simulink model "TosAeb"  (R2021a)
settings: Solver=FixedStepDiscrete  StartTime=0.0  StopTime=inf  FixedStep=0.01  RelTol=1e-3
undefined symbols (not defined in the file or in its folder): ACCModSts, AccSts1, AEB_ObjectMovingOrientation, AEB_ObjectMovingState, CN_BTCActive, CN_CurrentGearR, CN_CurrentGearR_DHT, CN_PTCActive, CN_VDCActive, CN_VSC_EspOff, CN_VSC_FCW_AEB_Error, CN_VSC_FCW_AEB_NoDispay, CN_VSC_FCW_AEB_PerfmDgdtn, CN_VSC_FCW_AEB_TempUnAvlbl, CN_VSC_VehStandstill, CNaccelAvoidMax, CNaccelAvoidMin, CNbrakePedalPress, CNcomfortStrLeftAccelMax, CNcomfortStrLeftAccelMin, CNcomfortStrRightAccelMax, CNcomfortStrRightAccelMin, CNDrvDoorOpen, CNDrvModeEco, CNDrvModeNorm, CNDrvModeOther, CNDrvModeSport, CNDrvSBRreminder, CNNull, CNobjInvalidID, CNPassDoorOpen, CNPassSBRreminder, CNPEBSAccelNegInf, CNPEBSAccelNull, CNPEBSTimeInf, CNPEBSTimeNull, CNSTOPMAX, CNSTOPNULL, CNsubjWidth, CNSwtOFF, CNSwtON, CNTTCMAX, CNTTCNULL, CNVehConfigAEBCycNone, CNVehConfigAEBNone, CNVehConfigAEBPedNone, CNVehConfigFCWNone, CNVehConfigJANone, CollisionSeverity, FALSE, FCWSnvty, JACycObjCalcInfoDefaultParams, JAPedObjCalcInfoDefaultParams, JAVehObjCalcInfoDefaultParams, latCycObjCalcInfoDefaultParams, latPedObjCalcInfoDefaultParams, longObjCalcInfoDefaultParams, ModSts, movingStatus, ObjectType ... (+1112)
libraries: simulink x3031, AEBSFunctionLib x50, Ta_Library x33, AEBSStateMachineLib x23, Mylibrary x12, simulink_extras x7, ObjSelect_lib x3, dspstat3 x2
(part 95 of 102)

== matlab function TosAeb/AEB_Objsel/TA_Processor/PreProcessor/ObjectPrecessor/TAObjInfoCalc/For Each Subsystem/Info_Check1/Orientation_check/Orientation_Classify ==
  data: ID_Change input; Fus_ObjOrientation output; Fus_ObsType input; Obj_Movingstate input; ObsID_u8 input; ObsMotionCategory_u8 input; ObsPedOrientation_u8 input; ObsPosX_s input; ObsPosY_s input; ObsVelY_s input; ObsVelXAbs_s input; ObsVelYAbs_s input; ObsCutInFlg_u8 input; ObsCutInSpd_s input; ObsCutInSpdValid_b input; ObsAngle_s input; ObsPosType_u8 input; ObsPosYDelay input; ObsSpdSqrt input; Obs_Vyaverage input
function Fus_ObjOrientation = fcn(ID_Change,Fus_ObsType,Obj_Movingstate,ObsID_u8,ObsMotionCategory_u8,ObsPedOrientation_u8,ObsPosX_s,ObsPosY_s,ObsVelY_s,...
    ObsVelXAbs_s,ObsVelYAbs_s,ObsCutInFlg_u8,ObsCutInSpd_s,ObsCutInSpdValid_b,ObsAngle_s,ObsPosType_u8,ObsPosYDelay,ObsSpdSqrt,Obs_Vyaverage)
%Fus_ObjOrientation 目标运动方向，定义如下
% 0：unknown 1:Preceeding 2:Crossing_to_Left
% 3: Crossing_to_Right 4:Cut_in 5:Cut_Out
% 6: Oncoming 7: LTAP 8:RTAP
ObjOrientation = uint8(0);
%检查目标是否为有效目标
if ID_Change == true || ObsID_u8 == uint8(0) ||Obj_Movingstate == uint8(0)||Obj_Movingstate == uint8(2)||Obj_Movingstate == uint8(3)
    ObjOrientation = uint8(0);
else
    %目标类型为车辆时目标的运动方向划分
    if Fus_ObsType > uint8(0) && Fus_ObsType < uint8(9)
        %Preceding判断
        if ObsPosX_s > single(0) && abs(ObsPosY_s) <= single(10.5) && ObsVelXAbs_s >= single(-0.5) && abs(ObsVelYAbs_s) <= (abs(ObsVelXAbs_s)+0.5) && (abs(ObsAngle_s)<single(pi/4)||abs(ObsAngle_s)>single(0.75*pi)) &&...
                ((ObsMotionCategory_u8 >= uint8(2) && ObsMotionCategory_u8 <= uint8(7))|| ObsMotionCategory_u8 == uint8(12))&& ObsPosType_u8>= uint8(1) && ObsPosType_u8<= uint8(3)
            %cut_in cut_out 判断
            if (ObsMotionCategory_u8 == uint8(5)||ObsMotionCategory_u8 == uint8(6)) && ObsCutInFlg_u8 == uint8(1) && abs(ObsCutInSpd_s) > single(0) && ObsCutInSpdValid_b == true && abs(ObsVelYAbs_s)>single(0)
                ObjOrientation = uint8(4);
            elseif ObsMotionCategory_u8 == uint8(7) && abs(ObsPosYDelay) - abs(ObsPosY_s) > single(0)
                ObjOrientation = uint8(5);
            else
                ObjOrientation = uint8(1);
            end
            % Crossing_to_Left/Right判断
        elseif ObsPosX_s > single(0) && ObsVelYAbs_s>= single(0) && abs(ObsVelYAbs_s) >= abs((ObsVelXAbs_s)+0.5) && (abs(ObsAngle_s)>single(pi/4)&&abs(ObsAngle_s)<single(0.75*pi))&& ObsMotionCategory_u8 == uint8(12)
            if ObsVelY_s > single(0) && ObsPosType_u8 ~= uint8(7) && ObsPosYDelay - ObsPosY_s< single(0)
                ObjOrientation = uint8(2);
            elseif ObsVelY_s < single(0) && ObsPosType_u8 ~= uint8(8) && ObsPosYDelay - ObsPosY_s> single(0)
                ObjOrientation = uint8(3);
            end
            % Oncoming
        elseif ObsPosX_s > single(0) && abs(ObsPosY_s) <= single(10.5) && ObsVelXAbs_s<single(0) && abs(ObsVelYAbs_s) <= abs(ObsVelXAbs_s) && (abs(ObsAngle_s)<single(pi/4)||abs(ObsAngle_s)>single(0.75*pi)) &&...
                ObsMotionCategory_u8 == uint8(13) && ObsPosType_u8 >= uint8(4) && ObsPosType_u8<= uint8(6)
            ObjOrientation = uint8(6);
            % LTAP
        elseif ObsMotionCategory_u8 == uint8(9)
            ObjOrientation = uint8(7);
            % RTAP
        elseif ObsMotionCategory_u8 == uint8(10)
            ObjOrientation = uint8(8);
        else
            ObjOrientation = uint8(0);
        end
        %二轮车运动方向判断
    elseif Fus_ObsType == uint8(9)||Fus_ObsType == uint8(10)
        if ObsPosX_s > single(0) && abs(ObsPosY_s) <= single(10.5) && ObsVelXAbs_s >= single(-0.5) && abs(ObsVelYAbs_s) <= (abs(ObsVelXAbs_s)+0.5) && (abs(ObsAngle_s)<single(pi/4)||abs(ObsAngle_s)>single(0.75*pi))
            %cut_in cut_out 判断
            if  abs(ObsVelYAbs_s)>single(0.5)&&abs(ObsVelYAbs_s)<single(2)&&ObsVelXAbs_s>ObsVelYAbs_s&&abs(ObsPosYDelay) - abs(ObsPosY_s) < single(0)
                ObjOrientation = uint8(4);
            elseif abs(ObsVelYAbs_s)>single(0.5)&&abs(ObsVelYAbs_s)<single(2)&&ObsVelXAbs_s>ObsVelYAbs_s&&abs(ObsPosYDelay) - abs(ObsPosY_s) > single(0)
                ObjOrientation = uint8(5);
            else
                ObjOrientation = uint8(1);
            end
            % Crossing_to_Left/Right判断
        elseif ObsPosX_s > single(0) && ObsVelYAbs_s>= single(0) && abs(ObsVelYAbs_s) >= abs((ObsVelXAbs_s)+0.5) && (abs(ObsAngle_s)>single(pi/4)&&abs(ObsAngle_s)<single(0.75*pi))
            if ObsVelY_s > single(0) && ObsPosYDelay - ObsPosY_s< single(0)
                ObjOrientation = uint8(3);
            elseif ObsVelY_s < single(0) && ObsPosYDelay - ObsPosY_s> single(0)
                ObjOrientation = uint8(2);
            end
            % Oncoming
        elseif ObsPosX_s > single(0) && abs(ObsPosY_s) <= single(10.5) && ObsVelXAbs_s<single(0) && abs(ObsVelYAbs_s) <= abs(ObsVelXAbs_s) && (abs(ObsAngle_s)<single(pi/4)||abs(ObsAngle_s)>single(0.75*pi))
            ObjOrientation = uint8(6);
            % LTAP
        elseif (abs(ObsAngle_s)>single(pi/4)&&abs(ObsAngle_s)<single(0.75*pi))&&ObsVelXAbs_s<single(-0.5)&&ObsVelYAbs_s<single(-0.5)
            ObjOrientation = uint8(7);
            % RTAP
        elseif (abs(ObsAngle_s)>single(pi/4)&&abs(ObsAngle_s)<single(0.75*pi))&&ObsVelXAbs_s<single(-0.5)&&ObsVelYAbs_s>single(-0.5)
            ObjOrientation = uint8(8);
        else
            ObjOrientation = uint8(0);
        end  
        %目标类型为行人或两轮车时目标的运动方向划分
    else
        if (ObsPedOrientation_u8 == uint8(1)||ObsPedOrientation_u8 == uint8(5)||ObsPedOrientation_u8 == uint8(8))&& ObsPosX_s > single(0)
            %Oncoming
            if ObsVelXAbs_s < single(-1) && abs(ObsVelYAbs_s) <= (abs(ObsVelXAbs_s)) && abs(ObsPosYDelay) - abs(ObsPosY_s) < single(1) && ObsSpdSqrt>=single(0.5)
                ObjOrientation = uint8(6);
                %Crossing_to_Left/Right判断
            elseif (abs(ObsVelYAbs_s) >= (abs(ObsVelXAbs_s)) || abs(ObsPosYDelay) - abs(ObsPosY_s) >= single(0.05)) && ObsVelY_s >single(0) && ObsPosYDelay - ObsPosY_s < single(0)&& abs(ObsVelY_s) >single(0.5)
                ObjOrientation = uint8(3);
            elseif (abs(ObsVelYAbs_s) >= (abs(ObsVelXAbs_s)) || abs(ObsPosYDelay) - abs(ObsPosY_s) >= single(0.05)) && ObsVelY_s <single(0) && ObsPosYDelay - ObsPosY_s > single(0)&& abs(ObsVelY_s) >single(0.5)
                ObjOrientation = uint8(2);
            elseif abs(ObsVelY_s) <= single(0.5)|| ObsSpdSqrt<=single(0.5)
                ObjOrientation = uint8(1);
            end
        elseif (ObsPedOrientation_u8 == uint8(2)||ObsPedOrientation_u8 == uint8(4)||ObsPedOrientation_u8 == uint8(7))&& ObsPosX_s > single(0)
            if ObsVelXAbs_s < single(-1) && abs(ObsVelYAbs_s) <= (abs(ObsVelXAbs_s)) && abs(ObsPosYDelay) - abs(ObsPosY_s) < single(0.05) && ObsSpdSqrt>=single(0.5)
                ObjOrientation = uint8(6);
                %Crossing_to_Left/Right判断
            elseif ObsVelXAbs_s > single(-0.5) && (abs(ObsVelYAbs_s) >= (abs(ObsVelXAbs_s)) || abs(ObsPosYDelay) - abs(ObsPosY_s) >= single(0.25)) && ObsVelY_s < single(0) && ObsPosYDelay - ObsPosY_s > single(0)
                ObjOrientation = uint8(3);
            elseif ObsVelXAbs_s > single(-0.5) && (abs(ObsVelYAbs_s) >= (abs(ObsVelXAbs_s)) || abs(ObsPosYDelay) - abs(ObsPosY_s) >= single(0.25)) && ObsVelY_s > single(0) && ObsPosYDelay - ObsPosY_s < single(0)
                ObjOrientation = uint8(2);
            elseif abs(ObsVelY_s) <= single(0.5)|| ObsSpdSqrt<=single(0.5)
                ObjOrientation = uint8(1);
            end
        elseif (ObsPedOrientation_u8 == uint8(3)||ObsPedOrientation_u8 == uint8(6))&& ObsPosX_s > single(0)
            if ObsVelXAbs_s < single(-1) && abs(ObsVelYAbs_s) <= (abs(ObsVelXAbs_s)) && abs(ObsPosYDelay) - abs(ObsPosY_s) < single(1) && ObsSpdSqrt>=single(0.5)
                ObjOrientation = uint8(6);
                %Crossing_to_Left/Right判断
            elseif(abs(ObsVelYAbs_s) >= (abs(ObsVelXAbs_s)) || abs(ObsPosYDelay) - abs(ObsPosY_s) >= single(0.05)) && ObsVelY_s < single(0) && ObsPosYDelay - ObsPosY_s > single(0) &&abs(Obs_Vyaverage)>single(0.5)
                ObjOrientation = uint8(3);
            elseif (abs(ObsVelYAbs_s) >= (abs(ObsVelXAbs_s)) || abs(ObsPosYDelay) - abs(ObsPosY_s) >= single(0.05)) && ObsVelY_s > single(0) && ObsPosYDelay - ObsPosY_s < single(0) && abs(Obs_Vyaverage)>single(0.5)
                ObjOrientation = uint8(2);
            elseif abs(ObsVelY_s) <= abs(ObsVelXAbs_s)|| ObsSpdSqrt<=single(0.5)
                ObjOrientation = uint8(1);
            end
        else
            ObjOrientation = uint8(0);
        end
    end
end
Fus_ObjOrientation = ObjOrientation;
end

== matlab function TosAeb/AEB_Objsel/TA_Processor/PreProcessor/ObjectPrecessor/TAObjInfoCalc/For Each Subsystem/Info_Check1/Orientation_check/Orientation_Classify2 ==
  data: ID_Change input; Fus_ObjOrientation output; Fus_ObsType input; ObsID_u8 input; ObsMotionCategory_u8 input; ObsPedOrientation_u8 input; ObsPosX_s input; ObsPosY_s input; ObsVelY_s input; ObsVelXAbs_s input; ObsVelYAbs_s input; ObsCutInFlg_u8 input; ObsCutInSpd_s input; ObsCutInSpdValid_b input; ObsAngle_s input; ObsPosType_u8 input; ObsPosYDelay input; ObsSpdSqrt input
function Fus_ObjOrientation = fcn(ID_Change,Fus_ObsType,ObsID_u8,ObsMotionCategory_u8,ObsPedOrientation_u8,ObsPosX_s,ObsPosY_s,ObsVelY_s,...
    ObsVelXAbs_s,ObsVelYAbs_s,ObsCutInFlg_u8,ObsCutInSpd_s,ObsCutInSpdValid_b,ObsAngle_s,ObsPosType_u8,ObsPosYDelay,ObsSpdSqrt)
%Fus_ObjOrientation 目标运动方向，定义如下
% 0：unknown 1:Preceeding 2:Crossing_to_Left
% 3: Crossing_to_Right 4:Cut_in 5:Cut_Out
% 6: Oncoming 7: LTAP 8:RTAP
ObjOrientation = uint8(0);
%检查目标是否为有效目标
if ID_Change == true || ObsID_u8 == uint8(0)
    ObjOrientation = uint8(0);
else
    %目标类型为车辆时目标的运动方向划分
    if Fus_ObsType > uint8(0) && Fus_ObsType < uint8(9)
        %Preceding判断
        if ObsPosX_s > single(0) && abs(ObsPosY_s) <= single(10.5) && ObsVelXAbs_s >= single(-0.5) && abs(ObsVelYAbs_s) <= (abs(ObsVelXAbs_s)+0.5) && (abs(ObsAngle_s)<single(pi/4)||abs(ObsAngle_s)>single(0.75*pi)) &&...
                ((ObsMotionCategory_u8 >= uint8(2) && ObsMotionCategory_u8 <= uint8(7))|| ObsMotionCategory_u8 == uint8(12))&& ObsPosType_u8>= uint8(1) && ObsPosType_u8<= uint8(3)
            %cut_in cut_out 判断
            if (ObsMotionCategory_u8 == uint8(5)||ObsMotionCategory_u8 == uint8(6)) && ObsCutInFlg_u8 == uint8(1) && abs(ObsCutInSpd_s) > single(0) && ObsCutInSpdValid_b == true && abs(ObsVelYAbs_s)>single(0)
                ObjOrientation = uint8(4);
            elseif ObsMotionCategory_u8 == uint8(7) && abs(ObsPosYDelay) - abs(ObsPosY_s) > single(0)
                ObjOrientation = uint8(5);
            else
                ObjOrientation = uint8(1);
            end
            % Crossing_to_Left/Right判断
        elseif ObsPosX_s > single(0) && abs(ObsVelYAbs_s) >= single(0) && abs(ObsVelYAbs_s) >= abs((ObsVelXAbs_s)+0.5) && (abs(ObsAngle_s)>single(pi/4)&&abs(ObsAngle_s)<single(0.75*pi))&& ObsMotionCategory_u8 == uint8(12)
            if ObsVelY_s > single(0) && ObsPosType_u8 ~= uint8(7) && ObsPosYDelay - ObsPosY_s< single(0)
                ObjOrientation = uint8(2);
            elseif ObsVelY_s < single(0) && ObsPosType_u8 ~= uint8(8) && ObsPosYDelay - ObsPosY_s> single(0)
                ObjOrientation = uint8(3);
            end
            % Oncoming
        elseif ObsPosX_s > single(0) && abs(ObsPosY_s) <= single(10.5) && ObsVelXAbs_s<single(0) && abs(ObsVelYAbs_s) <= abs(ObsVelXAbs_s) && (abs(ObsAngle_s)<single(pi/4)||abs(ObsAngle_s)>single(0.75*pi)) &&...
                ObsMotionCategory_u8 == uint8(13) && ObsPosType_u8 >= uint8(4) && ObsPosType_u8<= uint8(6)
            ObjOrientation = uint8(6);
            % LTAP
        elseif ObsMotionCategory_u8 == uint8(9)
            ObjOrientation = uint8(7);
            % RTAP
        elseif ObsMotionCategory_u8 == uint8(10)
            ObjOrientation = uint8(8);
        else
            ObjOrientation = uint8(0);
        end
        %二轮车运动方向判断
    elseif Fus_ObsType == uint8(9)||Fus_ObsType == uint8(10)
        if ObsPosX_s > single(0) && abs(ObsPosY_s) <= single(10.5) && ObsVelXAbs_s >= single(-0.5) && abs(ObsVelYAbs_s) <= (abs(ObsVelXAbs_s)+0.5) && (abs(ObsAngle_s)<single(pi/4)||abs(ObsAngle_s)>single(0.75*pi))
            %cut_in cut_out 判断
            if  abs(ObsVelYAbs_s)>single(0.5)&&abs(ObsVelYAbs_s)<single(2)&&ObsVelXAbs_s>ObsVelYAbs_s&&abs(ObsPosYDelay) - abs(ObsPosY_s) < single(0)
                ObjOrientation = uint8(4);
            elseif abs(ObsVelYAbs_s)>single(0.5)&&abs(ObsVelYAbs_s)<single(2)&&ObsVelXAbs_s>ObsVelYAbs_s&&abs(ObsPosYDelay) - abs(ObsPosY_s) > single(0)
                ObjOrientation = uint8(5);
            else
                ObjOrientation = uint8(1);
            end
            % Crossing_to_Left/Right判断
        elseif ObsPosX_s > single(0) && abs(ObsVelYAbs_s) >= single(0) && abs(ObsVelYAbs_s) >= abs((ObsVelXAbs_s)+0.5) && abs(ObsAngle_s)>single(pi/4) && abs(ObsAngle_s)<single(0.75*pi)
            if ObsVelY_s > single(0) && ObsPosYDelay - ObsPosY_s< single(0)
                ObjOrientation = uint8(2);
            elseif ObsVelY_s < single(0) && ObsPosYDelay - ObsPosY_s> single(0)
                ObjOrientation = uint8(3);
            end
            % Oncoming
        elseif ObsPosX_s > single(0) && abs(ObsPosY_s) <= single(10.5) && ObsVelXAbs_s<single(0) && abs(ObsVelYAbs_s) <= abs(ObsVelXAbs_s) && (abs(ObsAngle_s)<single(pi/4)||abs(ObsAngle_s)>single(0.75*pi))
            ObjOrientation = uint8(6);
            % LTAP
        elseif (abs(ObsAngle_s)>single(pi/4)&&abs(ObsAngle_s)<single(0.75*pi))&&ObsVelXAbs_s<single(-0.5)&&ObsVelYAbs_s<single(-0.5)
            ObjOrientation = uint8(7);
            % RTAP
        elseif (abs(ObsAngle_s)>single(pi/4)&&abs(ObsAngle_s)<single(0.75*pi))&&ObsVelXAbs_s<single(-0.5)&&ObsVelYAbs_s>single(-0.5)
            ObjOrientation = uint8(8);
        else
            ObjOrientation = uint8(0);
        end  
        %目标类型为行人或两轮车时目标的运动方向划分
    else
        if (ObsPedOrientation_u8 == uint8(1)||ObsPedOrientation_u8 == uint8(5)||ObsPedOrientation_u8 == uint8(8))&& ObsPosX_s > single(0)
            %Oncoming
            if ObsVelXAbs_s < single(-1) && abs(ObsVelYAbs_s) <= (abs(ObsVelXAbs_s)+single(0.5)) && abs(ObsPosYDelay) - abs(ObsPosY_s) < single(1) && ObsSpdSqrt>=single(0.5)
                ObjOrientation = uint8(6);
                %Crossing_to_Left/Right判断
            elseif ObsVelXAbs_s > single(-0.5) && (abs(ObsVelYAbs_s) >= (abs(ObsVelXAbs_s)+single(0.5)) || abs(ObsPosYDelay) - abs(ObsPosY_s) >= single(1)) && ObsVelY_s >single(0) && ObsPosYDelay - ObsPosY_s < single(0)&& abs(ObsVelY_s) >single(0.5)
                ObjOrientation = uint8(2);
            elseif ObsVelXAbs_s > single(-0.5) && (abs(ObsVelYAbs_s) >= (abs(ObsVelXAbs_s)+single(0.5)) || abs(ObsPosYDelay) - abs(ObsPosY_s) >= single(1)) && ObsVelY_s <single(0) && ObsPosYDelay - ObsPosY_s > single(0)&& abs(ObsVelY_s) >single(0.5)
                ObjOrientation = uint8(3);
            elseif abs(ObsVelY_s) <= single(0.5)|| ObsSpdSqrt<=single(0.5)
                ObjOrientation = uint8(1);
            end
        elseif (ObsPedOrientation_u8 == uint8(2)||ObsPedOrientation_u8 == uint8(4)||ObsPedOrientation_u8 == uint8(7))&& ObsPosX_s > single(0)
            if ObsVelXAbs_s < single(-1) && abs(ObsVelYAbs_s) <= (abs(ObsVelXAbs_s)+single(0.5)) && abs(ObsPosYDelay) - abs(ObsPosY_s) < single(1) && ObsSpdSqrt>=single(0.5)
                ObjOrientation = uint8(6);
                %Crossing_to_Left/Right判断
            elseif ObsVelXAbs_s > single(-0.5) && (abs(ObsVelYAbs_s) >= (abs(ObsVelXAbs_s)+single(0.5)) || abs(ObsPosYDelay) - abs(ObsPosY_s) >= single(1)) && ObsVelY_s >single(0) && ObsPosYDelay - ObsPosY_s < single(0)&& abs(ObsVelY_s) >single(0.5)
                ObjOrientation = uint8(2);
            elseif ObsVelXAbs_s > single(-0.5) && (abs(ObsVelYAbs_s) >= (abs(ObsVelXAbs_s)+single(0.5)) || abs(ObsPosYDelay) - abs(ObsPosY_s) >= single(1)) && ObsVelY_s <single(0) && ObsPosYDelay - ObsPosY_s > single(0)&& abs(ObsVelY_s) >single(0.5)
                ObjOrientation = uint8(3);
            elseif abs(ObsVelY_s) <= single(0.5)|| ObsSpdSqrt<=single(0.5)
                ObjOrientation = uint8(1);
            end
        elseif (ObsPedOrientation_u8 == uint8(3)||ObsPedOrientation_u8 == uint8(6))&& ObsPosX_s > single(0)
            if ObsVelXAbs_s < single(-1) && abs(ObsVelYAbs_s) <= (abs(ObsVelXAbs_s)+single(0.5)) && abs(ObsPosYDelay) - abs(ObsPosY_s) < single(1) && ObsSpdSqrt>=single(0.5)
                ObjOrientation = uint8(6);
                 %Crossing_to_Left/Right判断
            elseif ObsVelXAbs_s > single(-0.5) && (abs(ObsVelYAbs_s) >= (abs(ObsVelXAbs_s)+single(0.5)) || abs(ObsPosYDelay) - abs(ObsPosY_s) >= single(1)) && ObsVelY_s >single(0) && ObsPosYDelay - ObsPosY_s < single(0)&& abs(ObsVelY_s) >single(0.5)
                ObjOrientation = uint8(2);
            elseif ObsVelXAbs_s > single(-0.5) && (abs(ObsVelYAbs_s) >= (abs(ObsVelXAbs_s)+single(0.5)) || abs(ObsPosYDelay) - abs(ObsPosY_s) >= single(1)) && ObsVelY_s <single(0) && ObsPosYDelay - ObsPosY_s > single(0)&& abs(ObsVelY_s) >single(0.5)
                ObjOrientation = uint8(3);
            elseif abs(ObsVelY_s) <= single(0.5)|| ObsSpdSqrt<=single(0.5)
                ObjOrientation = uint8(1);
            end
        else
            ObjOrientation = uint8(0);
        end
    end
end
Fus_ObjOrientation = ObjOrientation;
end

== matlab function TosAeb/AEB_Objsel/TA_Processor/PreProcessor/ObjectPrecessor/TAObjInfoCalc/For Each Subsystem/Info_Check1/Orientation_check/Orientation_Classify3 ==
  data: ID_Change input; Fus_ObjOrientation output; Fus_ObsType input; ObsID_u8 input; ObsMotionCategory_u8 input; ObsPedOrientation_u8 input; ObsPosX_s input; ObsPosY_s input; ObsVelY_s input; ObsVelXAbs_s input; ObsVelYAbs_s input; ObsCutInFlg_u8 input; ObsCutInSpd_s input; ObsCutInSpdValid_b input; ObsAngle_s input; ObsPosType_u8 input; ObsPosYDelay input; ObsSpdSqrt input
function Fus_ObjOrientation = fcn(ID_Change,Fus_ObsType,ObsID_u8,ObsMotionCategory_u8,ObsPedOrientation_u8,ObsPosX_s,ObsPosY_s,ObsVelY_s,...
    ObsVelXAbs_s,ObsVelYAbs_s,ObsCutInFlg_u8,ObsCutInSpd_s,ObsCutInSpdValid_b,ObsAngle_s,ObsPosType_u8,ObsPosYDelay,ObsSpdSqrt)
%Fus_ObjOrientation 目标运动方向，定义如下
% 0：unknown 1:Preceeding 2:Crossing_to_Left
% 3: Crossing_to_Right 4:Cut_in 5:Cut_Out
% 6: Oncoming 7: LTAP 8:RTAP
ObjOrientation = uint8(0);
%检查目标是否为有效目标
if ID_Change == true || ObsID_u8 == uint8(0)
    ObjOrientation = uint8(0);
else
    %目标类型为车辆时目标的运动方向划分
    if Fus_ObsType > uint8(0) && Fus_ObsType < uint8(9)
        %Preceding判断
        if ObsPosX_s > single(0) && abs(ObsPosY_s) <= single(10.5) && ObsVelXAbs_s >= single(-0.5) && abs(ObsVelYAbs_s) <= (abs(ObsVelXAbs_s)+0.5) && (abs(ObsAngle_s)<single(pi/4)||abs(ObsAngle_s)>single(0.75*pi)) &&...
                ((ObsMotionCategory_u8 >= uint8(2) && ObsMotionCategory_u8 <= uint8(7))|| ObsMotionCategory_u8 == uint8(12))
            %cut_in cut_out 判断
            if (ObsMotionCategory_u8 == uint8(5)||ObsMotionCategory_u8 == uint8(6)) && ObsCutInFlg_u8 == uint8(1) && abs(ObsCutInSpd_s) > single(0) && ObsCutInSpdValid_b == true && abs(ObsVelYAbs_s)>single(0)
                ObjOrientation = uint8(4);
            elseif ObsMotionCategory_u8 == uint8(7) && abs(ObsPosYDelay) - abs(ObsPosY_s) > single(0)
                ObjOrientation = uint8(5);
            else
                ObjOrientation = uint8(1);
            end
            % Crossing_to_Left/Right判断
        elseif ObsPosX_s > single(0) && abs(ObsVelYAbs_s) >= single(0) && abs(ObsVelYAbs_s) >= abs((ObsVelXAbs_s)+0.5) && (abs(ObsAngle_s)>single(pi/4)&&abs(ObsAngle_s)<single(0.75*pi))&& ObsMotionCategory_u8 == uint8(12)
            if ObsVelY_s > single(0) && ObsPosType_u8 ~= uint8(7) && ObsPosYDelay - ObsPosY_s< single(0)
                ObjOrientation = uint8(2);
            elseif ObsVelY_s < single(0) && ObsPosType_u8 ~= uint8(8) && ObsPosYDelay - ObsPosY_s> single(0)
                ObjOrientation = uint8(3);
            end
            % Oncoming
        elseif ObsPosX_s > single(0) && abs(ObsPosY_s) <= single(10.5) && ObsVelXAbs_s<single(0) && abs(ObsVelYAbs_s) <= abs(ObsVelXAbs_s) && (abs(ObsAngle_s)<single(pi/4)||abs(ObsAngle_s)>single(0.75*pi)) &&...
                ObsMotionCategory_u8 == uint8(13) && ObsPosType_u8 >= uint8(4) && ObsPosType_u8<= uint8(6)
            ObjOrientation = uint8(6);
            % LTAP
        elseif ObsMotionCategory_u8 == uint8(9)
            ObjOrientation = uint8(7);
            % RTAP
        elseif ObsMotionCategory_u8 == uint8(10)
            ObjOrientation = uint8(8);
        else
            ObjOrientation = uint8(0);
        end
        %二轮车运动方向判断
    elseif Fus_ObsType == uint8(9)||Fus_ObsType == uint8(10)
        if ObsPosX_s > single(0) && abs(ObsPosY_s) <= single(10.5) && ObsVelXAbs_s >= single(-0.5) && abs(ObsVelYAbs_s) <= (abs(ObsVelXAbs_s)+0.5) && (abs(ObsAngle_s)<single(pi/4)||abs(ObsAngle_s)>single(0.75*pi))
            %cut_in cut_out 判断
            if  abs(ObsVelYAbs_s)>single(0.5)&&abs(ObsVelYAbs_s)<single(2)&&ObsVelXAbs_s>ObsVelYAbs_s&&abs(ObsPosYDelay) - abs(ObsPosY_s) < single(0)
                ObjOrientation = uint8(4);
            elseif abs(ObsVelYAbs_s)>single(0.5)&&abs(ObsVelYAbs_s)<single(2)&&ObsVelXAbs_s>ObsVelYAbs_s&&abs(ObsPosYDelay) - abs(ObsPosY_s) > single(0)
                ObjOrientation = uint8(5);
            else
                ObjOrientation = uint8(1);
            end
            % Crossing_to_Left/Right判断
        elseif ObsPosX_s > single(0) % && abs(ObsVelYAbs_s) >= single(0) && abs(ObsVelYAbs_s) >= abs((ObsVelXAbs_s)+0.5) && abs(ObsAngle_s)>single(pi/4) && abs(ObsAngle_s)<single(0.75*pi) % 20240125
            if ObsVelXAbs_s > single(-0.5) && (abs(ObsVelYAbs_s) >= (abs(ObsVelXAbs_s)+single(0.5)) || abs(ObsPosYDelay) - abs(ObsPosY_s) >= single(0.05)) && ObsVelY_s >single(0) && ObsPosYDelay - ObsPosY_s < single(0)&& abs(ObsVelY_s) >single(0.5)
                %ObsVelY_s > single(0) && ObsPosYDelay - ObsPosY_s<single(0） 
                ObjOrientation = uint8(2);
            elseif ObsVelXAbs_s > single(-0.5) && (abs(ObsVelYAbs_s) >= (abs(ObsVelXAbs_s)+single(0.5)) || abs(ObsPosYDelay) - abs(ObsPosY_s) >= single(0.05)) && ObsVelY_s <single(0) && ObsPosYDelay - ObsPosY_s > single(0)&& abs(ObsVelY_s) >single(0.5)
                %ObsVelY_s < single(0) && ObsPosYDelay - ObsPosY_s> single(0)
                ObjOrientation = uint8(3);
            end
            % Oncoming
        elseif ObsPosX_s > single(0) && abs(ObsPosY_s) <= single(10.5) && ObsVelXAbs_s<single(0) && abs(ObsVelYAbs_s) <= abs(ObsVelXAbs_s) && (abs(ObsAngle_s)<single(pi/4)||abs(ObsAngle_s)>single(0.75*pi))
            ObjOrientation = uint8(6);
            % LTAP
        elseif (abs(ObsAngle_s)>single(pi/4)&&abs(ObsAngle_s)<single(0.75*pi))&&ObsVelXAbs_s<single(-0.5)&&ObsVelYAbs_s<single(-0.5)
            ObjOrientation = uint8(7);
            % RTAP
        elseif (abs(ObsAngle_s)>single(pi/4)&&abs(ObsAngle_s)<single(0.75*pi))&&ObsVelXAbs_s<single(-0.5)&&ObsVelYAbs_s>single(-0.5)
            ObjOrientation = uint8(8);
        else
            ObjOrientation = uint8(0);
        end  
        %目标类型为行人或两轮车时目标的运动方向划分
    else
        if (ObsPedOrientation_u8 == uint8(1)||ObsPedOrientation_u8 == uint8(5)||ObsPedOrientation_u8 == uint8(8))&& ObsPosX_s > single(0)
            %Oncoming
            if ObsVelXAbs_s < single(-1) && abs(ObsVelYAbs_s) <= (abs(ObsVelXAbs_s)+single(0.5)) && abs(ObsPosYDelay) - abs(ObsPosY_s) < single(1) && ObsSpdSqrt>=single(0.5)
                ObjOrientation = uint8(6);
                %Crossing_to_Left/Right判断
            elseif ObsVelXAbs_s > single(-0.5) && (abs(ObsVelYAbs_s) >= (abs(ObsVelXAbs_s)+single(0.5))&& ObsVelY_s >single(0))          % || abs(ObsPosYDelay) - abs(ObsPosY_s) >= single(0.5)) && ObsVelY_s >single(0) && ObsPosYDelay - ObsPosY_s < single(0)&& abs(ObsVelY_s) >single(0.5)
                ObjOrientation = uint8(2);
            elseif ObsVelXAbs_s > single(-0.5) && (abs(ObsVelYAbs_s) >= (abs(ObsVelXAbs_s)+single(0.5))&&ObsVelY_s <single(0))             %|| abs(ObsPosYDelay) - abs(ObsPosY_s) <= single(0.5)) && ObsVelY_s <single(0) && ObsPosYDelay - ObsPosY_s > single(0)&& abs(ObsVelY_s) >single(0.5)
                ObjOrientation = uint8(3);
            elseif abs(ObsVelY_s) <= single(0.5)|| ObsSpdSqrt<=single(0.5)
                ObjOrientation = uint8(1);
            end
        elseif (ObsPedOrientation_u8 == uint8(2)||ObsPedOrientation_u8 == uint8(4)||ObsPedOrientation_u8 == uint8(7))&& ObsPosX_s > single(0)
            if ObsVelXAbs_s < single(-1) && abs(ObsVelYAbs_s) <= (abs(ObsVelXAbs_s)+single(0.5)) && abs(ObsPosYDelay) - abs(ObsPosY_s) < single(1) && ObsSpdSqrt>=single(0.5)
                ObjOrientation = uint8(6);
                %Crossing_to_Left/Right判断
            elseif ObsVelXAbs_s > single(-0.5) && (abs(ObsVelYAbs_s) >= (abs(ObsVelXAbs_s)+single(0.5))&& ObsVelY_s >single(0)) %      || abs(ObsPosYDelay) - abs(ObsPosY_s) >= single(1)) && ObsVelY_s >single(0) && ObsPosYDelay - ObsPosY_s < single(0)&& abs(ObsVelY_s) >single(0.5)
                ObjOrientation = uint8(2);
            elseif ObsVelXAbs_s > single(-0.5) && (abs(ObsVelYAbs_s) >= (abs(ObsVelXAbs_s)+single(0.5))&& ObsVelY_s <single(0)) %      || abs(ObsPosYDelay) - abs(ObsPosY_s) >= single(1)) && ObsVelY_s <single(0) && ObsPosYDelay - ObsPosY_s > single(0)&& abs(ObsVelY_s) >single(0.5)
                ObjOrientation = uint8(3);
            elseif abs(ObsVelY_s) <= single(0.5)|| ObsSpdSqrt<=single(0.5)
                ObjOrientation = uint8(1);
            end
        elseif (ObsPedOrientation_u8 == uint8(3)||ObsPedOrientation_u8 == uint8(6))&& ObsPosX_s > single(0)
            if ObsVelXAbs_s < single(-1) && abs(ObsVelYAbs_s) <= (abs(ObsVelXAbs_s)+single(0.5)) && abs(ObsPosYDelay-ObsPosY_s) < single(0.5) && ObsSpdSqrt>=single(0.5)
                ObjOrientation = uint8(6);
                 %Crossing_to_Left/Right判断
            elseif ObsVelXAbs_s > single(-0.5) && (abs(ObsVelYAbs_s) >= (abs(ObsVelXAbs_s)+single(0.5)) && ObsVelY_s >single(0))
            %elseif ObsVelXAbs_s > single(-0.5) && (abs(ObsVelYAbs_s) >= (abs(ObsVelXAbs_s)+single(0.5)) || abs(ObsPosYDelay-ObsPosY_s) >= single(1)) && ObsVelY_s >single(0) && ObsPosYDelay - ObsPosY_s < single(0)&& abs(ObsVelY_s) >single(0.5)
                ObjOrientation = uint8(2);
            
            %elseif ObsVelXAbs_s > single(-0.5) && (abs(ObsVelYAbs_s) >= (abs(ObsVelXAbs_s)+single(0.5)) || abs(ObsPosYDelay- ObsPosY_s) >= single(1)) && ObsVelY_s <single(0) && ObsPosYDelay - ObsPosY_s > single(0)&& abs(ObsVelY_s) >single(0.5)
             elseif ObsVelXAbs_s > single(-0.5) && (abs(ObsVelYAbs_s) >= (abs(ObsVelXAbs_s)+single(0.5)) && ObsVelY_s <single(0))
            ObjOrientation = uint8(3);
            elseif abs(ObsVelY_s) <= single(0.5)|| ObsSpdSqrt<=single(0.5)
                ObjOrientation = uint8(1);
            end
        else
            ObjOrientation = uint8(0);
        end
    end
end
Fus_ObjOrientation = ObjOrientation;
end

== matlab function TosAeb/AEB_Objsel/TA_Processor/PreProcessor/ObjectPrecessor/TAObjInfoCalc/For Each Subsystem/Info_Check1/State_check/State_Classify1 ==
  data: ID_Change input; Fus_ObjState output; Fus_ObsType input; ObsID_u8 input; ObsMeasureStatus_u8 input; ObsVelXAbs_s input; ObsVelYAbs_s input; Spd_Stay input
function Fus_ObjState = fcn(ID_Change, Fus_ObsType, ObsID_u8, ObsMeasureStatus_u8, ObsVelXAbs_s, ObsVelYAbs_s, Spd_Stay)

%Fus_ObjState 目标运动状态
% 0：unknown 1:Moving 2:Stationary
% 3: Stopped 4:Moving_Slowly
ObjState = uint8(0);
%判断目标ID是否有效
if ID_Change == true || ObsID_u8 == uint8(0)
    ObjState = uint8(0);
else
    %对目标类型为车辆的运动状态进行校核
    if Fus_ObsType > uint8(0) && Fus_ObsType < uint8(9)
        if ObsMeasureStatus_u8 == uint8(1)
            if abs(ObsVelXAbs_s) + abs(ObsVelYAbs_s) > single(2.5)
                ObjState = uint8(1);
            elseif abs(ObsVelXAbs_s) + abs(ObsVelYAbs_s) <= single(0.9) && Spd_Stay <= single(0.5)
                ObjState = uint8(2);
            elseif abs(ObsVelXAbs_s) + abs(ObsVelYAbs_s) <= single(0.9) && Spd_Stay > single(0.5)
                ObjState = uint8(3);
            elseif abs(ObsVelXAbs_s) + abs(ObsVelYAbs_s) >= single(0.9) && abs(ObsVelXAbs_s) + abs(ObsVelYAbs_s) <= single(2.5)
                ObjState = uint8(4);
            else
                ObjState = uint8(0);
            end
        elseif ObsMeasureStatus_u8 >=uint8(2) && ObsMeasureStatus_u8 <= uint8(5)
            ObjState = ObsMeasureStatus_u8-uint8(1);
        else
            ObjState = uint8(0);
        end
        %计算行人的运动状态
    else
        if abs(ObsVelXAbs_s) + abs(ObsVelYAbs_s) > single(2.5) 
             ObjState = uint8(1);
        elseif abs(ObsVelXAbs_s) + abs(ObsVelYAbs_s) <= single(0.9) && Spd_Stay <= single(0.5)
            ObjState = uint8(2);
        elseif abs(ObsVelXAbs_s) + abs(ObsVelYAbs_s) <= single(1) && Spd_Stay > single(0.5)
            ObjState = uint8(3);
        elseif abs(ObsVelXAbs_s) + abs(ObsVelYAbs_s) >= single(0.9) && abs(ObsVelXAbs_s) + abs(ObsVelYAbs_s) <= single(2.5)
            ObjState = uint8(4);
        else
            ObjState = uint8(0);
        end
    end
end
Fus_ObjState = ObjState;
end

== matlab function TosAeb/AEB_Objsel/TA_Processor/PreProcessor/ObjectPrecessor/TAObjInfoCalc/For Each Subsystem/Info_Check1/Type_Precheck/Type_comfig ==
  data: ID_Change input; ObsID_u8 input; Fus_ObjType output; ObsClass_u8 input; ObsVhSubType_u8 input; ObsPedSubType_u8 input; ObsPedPos_u8 input; ObsSpd_s input; P_TA_PrmForMotorSpdThred input
function Fus_ObjType = fcn(ID_Change,ObsID_u8,ObsClass_u8,ObsVhSubType_u8,ObsPedSubType_u8,ObsPedPos_u8,ObsSpd_s,P_TA_PrmForMotorSpdThred)
%Fus_ObjType 传感器输出目标类型，对应参数如下：
% 0: unknown  1: Bus  2: Small_Medium_Car (中小型轿车) 3: Trucks (大卡车)  4: Motors (三轮车)
% 5: Special_vehicle (特殊车) 6: Tiny_car (微型车) 7: Lorry (小货车) 8: MiniVan (面包车)
% 9: Motor Cyclist(摩托车) 10：Cyclist（自行车）11：Pedestrian(Adult) 12:Pedestrian（child）
% 13: others(动物或不能划分清楚的)
%判断目标的有效性，如果目标无效，目标类型为unknown
Fus_ObjType = uint8(0);
if ID_Change == true || ObsID_u8 == uint8(0)
    Fus_ObjType = uint8(0);
%当目标目标为车辆时
elseif ObsClass_u8 ~= uint8(2) && ObsClass_u8 ~= uint8(3)
        if ObsClass_u8 == uint8(1) && ObsVhSubType_u8==uint8(0)
          Fus_ObjType = uint8(13); 
        else
          Fus_ObjType = ObsVhSubType_u8;
        end
elseif ObsClass_u8 == uint8(2)
        if ObsPedSubType_u8 == uint8(0) || ObsPedPos_u8 == uint8(0)
            Fus_ObjType = uint8(13);
        elseif ObsPedSubType_u8==uint8(1)
            Fus_ObjType = uint8(11);
        elseif ObsPedSubType_u8==uint8(2)
            Fus_ObjType = uint8(12); 
        end
elseif ObsClass_u8 == uint8(3)
       if ObsSpd_s >= P_TA_PrmForMotorSpdThred
        Fus_ObjType = uint8(9);
       elseif ObsSpd_s < P_TA_PrmForMotorSpdThred
        Fus_ObjType = uint8(10);
       end
else 
    Fus_ObjType = uint8(0);
end   
end

== matlab function TosAeb/AEB_Objsel/TA_Processor/PreProcessor/ObjectPrecessor/TAObjInfoCalc/For Each Subsystem/Info_Check1/Type_Process/MATLAB Function2 ==
  data: C_ObjType output; Obj_type input; Obj_Length input; Obj_Width input; Obj_Height input; Obj_dx input; abs_Spd input; P_TA_ObjTruckLengthPara input; P_TA_ObjTruckWidthPara input; P_TA_ObjCarLengthPara input; P_TA_ObjCarWidthPara input; P_TA_ObjMotorBicycleLengthPara input; P_TA_ObjMotorBicycleWidthPara input; P_TA_ObjBicycleLengthPara input; P_TA_ObjBicycleWidthPara input; P_TA_ObjPedestrainLengthPara input; P_TA_ObjPedestrainWidthPara input; P_TA_ObjBusLengthPara input; P_TA_ObjBusWidthPara input; C_ObjLength output single; C_ObjWidth output single; C_ObjHight output; P_TA_ObjLongthValidFactor input; P_TA_ObjWidthValidFactor input; P_TA_ObjMotorVehSpdThd input; P_TA_ObjPedestrainSpdThd input; C_Objdx output
function [C_ObjType,C_ObjLength,C_ObjWidth,C_ObjHight,C_Objdx]  = fcn(Obj_type,Obj_Length,Obj_Width,Obj_Height,Obj_dx,abs_Spd,P_TA_ObjTruckLengthPara,P_TA_ObjTruckWidthPara,P_TA_ObjCarLengthPara,P_TA_ObjCarWidthPara,...
    P_TA_ObjMotorBicycleLengthPara,P_TA_ObjMotorBicycleWidthPara,P_TA_ObjBicycleLengthPara,P_TA_ObjBicycleWidthPara,P_TA_ObjPedestrainLengthPara,...
    P_TA_ObjPedestrainWidthPara,P_TA_ObjBusLengthPara,P_TA_ObjBusWidthPara,P_TA_ObjLongthValidFactor,P_TA_ObjWidthValidFactor,P_TA_ObjMotorVehSpdThd,P_TA_ObjPedestrainSpdThd)
%重新校验后目标类型如下：
%0x0: Unknwon 0x1: Bus 0x2: Car 0x3: Truck 0x4: Motor Bike
%0x5: Bicycle 0x6: Pedestrian 0x7: Animals 
C_ObjType = uint8(255);
C_ObjLength = single(0);
C_ObjWidth = single(0);
C_ObjHight = single(0);
C_Objdx = single(Obj_dx);
Bound_Vecter = single(zeros(1,6));
%目标存在时
if Obj_type ~= uint8(0)
    Bound_Vecter(1) = vecter_cal(Obj_Length,Obj_Width,P_TA_ObjTruckLengthPara,P_TA_ObjTruckWidthPara);
    Bound_Vecter(2) = vecter_cal(Obj_Length,Obj_Width,P_TA_ObjCarLengthPara,P_TA_ObjCarWidthPara);
    Bound_Vecter(3) = vecter_cal(Obj_Length,Obj_Width,P_TA_ObjBusLengthPara,P_TA_ObjBusWidthPara);
    Bound_Vecter(4) = vecter_cal(Obj_Length,Obj_Width,P_TA_ObjMotorBicycleLengthPara,P_TA_ObjMotorBicycleWidthPara);
    Bound_Vecter(5) = vecter_cal(Obj_Length,Obj_Width,P_TA_ObjBicycleLengthPara,P_TA_ObjBicycleWidthPara);
    Bound_Vecter(6) = vecter_cal(Obj_Length,Obj_Width,P_TA_ObjPedestrainLengthPara,P_TA_ObjPedestrainWidthPara);
    [~,I] = sort(Bound_Vecter);
    if Obj_type == uint8(3)
        %Truck相关数据处理
        if I(1) == uint8(1)
            C_ObjType = uint8(3);
            C_ObjLength = Obj_Length;
            C_ObjWidth = Obj_Width;
            C_ObjHight = Obj_Height;
            C_Objdx = Obj_dx;
        else
            [t_flg] = caul(Obj_Length,Obj_Width,P_TA_ObjTruckLengthPara,P_TA_ObjTruckWidthPara,P_TA_ObjLongthValidFactor,P_TA_ObjWidthValidFactor);
            C_Objdx = Obj_dx;
            if t_flg == true && Obj_Height < single(1.9)
                if abs_Spd >= P_TA_ObjMotorVehSpdThd 
                    C_ObjType = uint8(2);
                    C_ObjLength = P_TA_ObjCarLengthPara;
                    C_ObjWidth = P_TA_ObjCarWidthPara;
                    C_ObjHight = single(1.85);
                elseif abs_Spd < P_TA_ObjMotorVehSpdThd && abs_Spd >= P_TA_ObjPedestrainSpdThd 
                    C_ObjType = uint8(5);
                    C_ObjLength = P_TA_ObjBicycleLengthPara;
                    C_ObjWidth = P_TA_ObjBicycleWidthPara;
                    C_ObjHight = single(1.80);
                else
                    C_ObjType = uint8(6);
                    C_ObjLength = P_TA_ObjPedestrainLengthPara;
                    C_ObjWidth = P_TA_ObjPedestrainWidthPara;
                    C_ObjHight = single(1.75);
                end
            else
                C_ObjType = uint8(0);
                C_ObjLength = Obj_Length;
                C_ObjWidth = Obj_Width;
                C_ObjHight = Obj_Height;
            end
        end
    elseif Obj_type == uint8(2) || (Obj_type >= uint8(4) && Obj_type <= uint8(8))
        %Car相关数据处理
        if I(1) == uint8(2)
            C_ObjType = uint8(2);
            C_ObjLength = Obj_Length;
            C_ObjWidth = Obj_Width;
            C_ObjHight = Obj_Height;
        else
            [t_flg] = caul(Obj_Length,Obj_Width,P_TA_ObjCarLengthPara,P_TA_ObjCarWidthPara,P_TA_ObjLongthValidFactor,P_TA_ObjWidthValidFactor);
            C_Objdx = Obj_dx;
            if t_flg == true
                if abs_Spd >= P_TA_ObjMotorVehSpdThd
                    C_ObjType = uint8(2);
                    C_ObjLength = P_TA_ObjCarLengthPara;
                    C_ObjWidth = P_TA_ObjCarWidthPara;
                    C_ObjHight = single(1.85);
                elseif abs_Spd < P_TA_ObjMotorVehSpdThd && abs_Spd >= P_TA_ObjPedestrainSpdThd
                    C_ObjType = uint8(5);
                    C_ObjLength = P_TA_ObjBicycleLengthPara;
                    C_ObjWidth = P_TA_ObjBicycleWidthPara;
                    C_ObjHight = single(1.80);
                else
                    C_ObjType = uint8(6);
                    C_ObjLength = P_TA_ObjPedestrainLengthPara;
                    C_ObjWidth = P_TA_ObjPedestrainWidthPara;
                    C_ObjHight = single(1.75);
                end
            else
                C_ObjType = uint8(0);
                C_ObjLength = Obj_Length;
                C_ObjWidth = Obj_Width;
                C_ObjHight = Obj_Height;
            end
        end
        elseif Obj_type == uint8(1)
            %bus相关数据处理
            if I(1) == uint8(3)
                C_ObjType = uint8(1);
                C_ObjLength = Obj_Length;
                C_ObjWidth = Obj_Width;
                C_ObjHight = Obj_Height;
            else
                [t_flg] = caul(Obj_Length,Obj_Width,P_TA_ObjBusLengthPara,P_TA_ObjBusWidthPara,P_TA_ObjLongthValidFactor,P_TA_ObjWidthValidFactor);
                C_Objdx = Obj_dx;
                if t_flg == true
                    if abs_Spd >= P_TA_ObjMotorVehSpdThd
                        C_ObjType = uint8(2);
                        C_ObjLength = P_TA_ObjCarLengthPara;
                        C_ObjWidth = P_TA_ObjCarWidthPara;
                        C_ObjHight = single(1.85);
                    elseif abs_Spd < P_TA_ObjMotorVehSpdThd && abs_Spd >= P_TA_ObjPedestrainSpdThd
                        C_ObjType = uint8(5);
                        C_ObjLength = P_TA_ObjBicycleLengthPara;
                        C_ObjWidth = P_TA_ObjBicycleWidthPara;
                        C_ObjHight = single(1.80);
                    else
                        C_ObjType = uint8(6);
                        C_ObjLength = P_TA_ObjPedestrainLengthPara;
                        C_ObjWidth = P_TA_ObjPedestrainWidthPara;
                        C_ObjHight = single(1.75);
                    end
                else
                    C_ObjType = uint8(0);
                    C_ObjLength = Obj_Length;
                    C_ObjWidth = Obj_Width;
                    C_ObjHight = Obj_Height;
                end
            end
    elseif Obj_type == uint8(9)
        %Motor相关数据处理
        if I(1) == uint8(4)
            C_ObjType = uint8(4);
            C_ObjLength = Obj_Length;
            C_ObjWidth = Obj_Width;
            C_ObjHight = Obj_Height;
        else
            [t_flg] = caul(Obj_Length,Obj_Width,P_TA_ObjMotorBicycleLengthPara,P_TA_ObjMotorBicycleWidthPara,P_TA_ObjLongthValidFactor,P_TA_ObjWidthValidFactor);
            C_Objdx = Obj_dx;
            if t_flg == true
                if abs_Spd >= P_TA_ObjMotorVehSpdThd
                    C_ObjType = uint8(2);
                    C_ObjLength = P_TA_ObjCarLengthPara;
                    C_ObjWidth = P_TA_ObjCarWidthPara;
                    C_ObjHight = single(1.85);
                elseif abs_Spd < P_TA_ObjMotorVehSpdThd && abs_Spd >= P_TA_ObjPedestrainSpdThd
                    C_ObjType = uint8(5);
                    C_ObjLength = P_TA_ObjBicycleLengthPara;
                    C_ObjWidth = P_TA_ObjBicycleWidthPara;
                    C_ObjHight = single(1.80);
                else
                    C_ObjType = uint8(6);
                    C_ObjLength = P_TA_ObjPedestrainLengthPara;
                    C_ObjWidth = P_TA_ObjPedestrainWidthPara;
                    C_ObjHight = single(1.75);
                end
            else
                C_ObjType = uint8(0);
                C_ObjLength = Obj_Length;
                C_ObjWidth = Obj_Width;
                C_ObjHight = Obj_Height;
            end
        end
    elseif Obj_type == uint8(10)
        %Bicycle相关数据处理
        if I(1) == uint8(5)||(Obj_Length == single(0)&& Obj_Width == single(0) && abs(Obj_Height) ~= single(0))
            C_ObjType = uint8(5);
            C_ObjLength = P_TA_ObjBicycleLengthPara;
            C_ObjWidth = P_TA_ObjBicycleWidthPara;
             C_ObjHight = single(1.80);
        else
            [t_flg] = caul(Obj_Length,Obj_Width,P_TA_ObjBicycleLengthPara,P_TA_ObjBicycleWidthPara,P_TA_ObjLongthValidFactor,P_TA_ObjWidthValidFactor);
            C_Objdx = Obj_dx;
            if t_flg == true
                if abs_Spd >= P_TA_ObjMotorVehSpdThd
                    C_ObjType = uint8(2);
                    C_ObjLength = P_TA_ObjCarLengthPara;
                    C_ObjWidth = P_TA_ObjCarWidthPara;
                    C_ObjHight = single(1.85);
                elseif abs_Spd < P_TA_ObjMotorVehSpdThd && abs_Spd >= P_TA_ObjPedestrainSpdThd
                    C_ObjType = uint8(5);
                    C_ObjLength = P_TA_ObjBicycleLengthPara;
                    C_ObjWidth = P_TA_ObjBicycleWidthPara;
                    C_ObjHight = single(1.80);
                else
                    C_ObjType = uint8(6);
                    C_ObjLength = P_TA_ObjPedestrainLengthPara;
                    C_ObjWidth = P_TA_ObjPedestrainWidthPara;
                    C_ObjHight = single(1.75);
                end
            else
                C_ObjType = uint8(0);
                C_ObjLength = Obj_Length;
                C_ObjWidth = Obj_Width;
                C_ObjHight = Obj_Height;
            end
        end
    elseif Obj_type == uint8(11) || Obj_type == uint8(12)
        %Pedestrian相关数据处理
        if I(1) == uint8(6)||(Obj_Length == single(0)&& Obj_Width == single(0) && abs(Obj_Height) ~= single(0))
            C_ObjType = uint8(6);
            C_ObjLength = P_TA_ObjPedestrainLengthPara;
            C_ObjWidth = P_TA_ObjPedestrainWidthPara;
            C_ObjHight = Obj_Height;
        else
            [t_flg] = caul(Obj_Length,Obj_Width,P_TA_ObjPedestrainLengthPara,P_TA_ObjPedestrainWidthPara,P_TA_ObjLongthValidFactor,P_TA_ObjWidthValidFactor);
            C_Objdx = Obj_dx;
            if t_flg == true
                if abs_Spd >= P_TA_ObjMotorVehSpdThd
                    C_ObjType = uint8(2);
                    C_ObjLength = P_TA_ObjCarLengthPara;
                    C_ObjWidth = P_TA_ObjCarWidthPara;
                    C_ObjHight = single(1.85);
                elseif abs_Spd < P_TA_ObjMotorVehSpdThd && abs_Spd >= P_TA_ObjPedestrainSpdThd
                    C_ObjType = uint8(5);
                    C_ObjLength = P_TA_ObjBicycleLengthPara;
                    C_ObjWidth = P_TA_ObjBicycleWidthPara;
                    C_ObjHight = single(1.80);
                else
                    C_ObjType = uint8(6);
                    C_ObjLength = P_TA_ObjPedestrainLengthPara;
                    C_ObjWidth = P_TA_ObjPedestrainWidthPara;
                    C_ObjHight = single(1.75);
                end
            else
                C_ObjType = uint8(0);
                C_ObjLength = Obj_Length;
                C_ObjWidth = Obj_Width;
                C_ObjHight = Obj_Height;
            end
        end
    else
        C_ObjType = uint8(7);
        C_ObjLength = Obj_Length;
        C_ObjWidth = Obj_Width;
        C_ObjHight = Obj_Height;
    end    
end
end
%% 初步判断
function factor = vecter_cal(Obj_longth,Obj_Widths,PA_long,PA_width)
f_long = (Obj_longth - PA_long)/Obj_longth>0;
f_width = (Obj_Widths - PA_width)/Obj_Widths>0;
factor = f_long * f_width;
end
%% 对目标长宽进行校核
function [t_ChangeFlg] = caul(Obj_long,Obj_Width,P_long,P_width,P_longFactor,P_WidthFactor)
if Obj_long < single(0) || Obj_Width < single(0)
    t_ChangeFlg = true;
elseif  abs(Obj_long - P_long) > P_longFactor * Obj_long && abs(Obj_Width - P_width) > P_WidthFactor * Obj_Width
    t_ChangeFlg = true;
else
    t_ChangeFlg = false;
end
end
